simulink library "Renault_Library"  (Simulink 9.2)
settings: Solver=ode3  StartTime=0.0  StopTime=10.0  FixedStep=0.020  RelTol=1e-3  EnableMultiTasking=on
undefined symbols (not defined in the file or in its folder): ABC, AssystemProposal, b, chkval, CoeffA_C, CoeffB_C, CoeffMean_C, ConfTime_C, DiagDelay_C, DividePercent, FailureConfActive_C, FallingEdgeDuration_C, HighLevelThresholdVoltage_C, HighLevelVoltage_C, HighLevelVoltageTunable_C, InitValue_C, InputVoltRangeMax_C, InputVoltRangeMin_C, LowActiveContact_C, LowLevelThresholdVoltage_C, LowLevelVoltage_C, MaxOperatingVoltage_C, MinOperatingVoltage_C, mV2V_conversion, OUT1, OutOfRangeHigh_C, OutOfRangeLow_C, PinSupplySaturationMax, PinSupplySaturationMin, Res1Value_C, Res2Value_C, Res3Value_C, RisingEdgeDuration_C, RSA_CANSignalFailMonitoring, SampleTime_C, service14Table, service19Table, service22Table, service24Table, service28Table, service2ETable, service2FTable, service31Table, service87Table, SizeXStateDID2FTable, SizeXTable14, SizeXTable19, SizeXTable22, SizeXTable24, SizeXTable28, SizeXTable2E, SizeXTable2F, SizeXTable31, SizeXTable87, SizeYStateDID2FTable, SizeYTable14, SizeYTable19, SizeYTable22, SizeYTable24, SizeYTable28 ... (+22)
undefined symbols (not defined in the file): BatteryVoltage_ActivationThreshold_C, FrameStatus_AbsentValue_C, FrameStatus_ConfAbsentValue_C, MemThreshold_C, NAMEOFTHEMODEL_SAMPLE_TIME_CST, NbAbs1_C, NbConfAbs1_C, NbConfPres1_C, NbPres1_C, NOMDUSIGNAL_FaultConfTime_C, NOMDUSIGNAL_FaultRehabTime_C, NOMDUSIGNAL_switchvalue_C, NOMDUSIGNAL_unavailablevalue_C, PeriodFrame1_C, ResetTime_C, WakeUpTime_C
libraries: $bdroot x112, simulink x75
(part 23 of 32)

== system Renault_Library/Services ECU/Diag/RSA_DiagnosticManagement_v02.00/DIAGNOSTIC_SANCTION_TOP/Service27/SubFunction_Supported_Service27 ==
  DIAG_BUFFER_REQUEST : Inport
  BitwiseOperator1 : simulink/Logic and Bit Operations/Bitwise Operator  logicop=AND  UseBitMask=on  BitMask=bin2dec('01111111')  BitMaskRealWorld=Stored Integer
  BitwiseOperator4 : simulink/Logic and Bit Operations/Bitwise Operator  logicop=AND  UseBitMask=on  BitMask=bin2dec('10000000')  BitMaskRealWorld=Stored Integer
  Constant : Constant  OutDataTypeStr=Inherit: Inherit via back propagation  SampleTime=-1
  Constant1 : Constant  Value=2  OutDataTypeStr=Inherit: Inherit via back propagation  SampleTime=-1
  Constant10 : Constant  Value=RequestSeed5  OutDataTypeStr=Inherit: Inherit via back propagation  SampleTime=-1
  Constant{5,7,9,11,15,18,21,26,28,30} (10 alike) : Constant  Value=true  OutDataTypeStr=boolean  SampleTime=-1
  Constant12 : Constant  Value=65  OutDataTypeStr=Inherit: Inherit via back propagation  SampleTime=-1
  Constant13 : Constant  Value=4  OutDataTypeStr=Inherit: Inherit via back propagation  SampleTime=-1
  Constant14 : Constant  Value=SendKey8  OutDataTypeStr=Inherit: Inherit via back propagation  SampleTime=-1
  Constant16 : Constant  OutDataTypeStr=Inherit: Inherit via back propagation  SampleTime=-1
  Constant17 : Constant  Value=RequestSeed7  OutDataTypeStr=Inherit: Inherit via back propagation  SampleTime=-1
  Constant19 : Constant  Value=7  OutDataTypeStr=Inherit: Inherit via back propagation  SampleTime=-1
  Constant2 : Constant  Value=3  OutDataTypeStr=Inherit: Inherit via back propagation  SampleTime=-1
  Constant20 : Constant  Value=SendKey42  OutDataTypeStr=Inherit: Inherit via back propagation  SampleTime=-1
  Constant22 : Constant  Value=66  OutDataTypeStr=Inherit: Inherit via back propagation  SampleTime=-1
  Constant23 : Constant  Value=6  OutDataTypeStr=Inherit: Inherit via back propagation  SampleTime=-1
  Constant24 : Constant  Value=RequestSeed41  OutDataTypeStr=Inherit: Inherit via back propagation  SampleTime=-1
  Constant25 : Constant  Value=8  OutDataTypeStr=Inherit: Inherit via back propagation  SampleTime=-1
  Constant27 : Constant  Value=SendKey4  OutDataTypeStr=Inherit: Inherit via back propagation  SampleTime=-1
  Constant29 : Constant  Value=SendKey6  OutDataTypeStr=Inherit: Inherit via back propagation  SampleTime=-1
  Constant3 : Constant  Value=RequestSeed  OutDataTypeStr=Inherit: Inherit via back propagation  SampleTime=-1
  Constant4 : Constant  Value=5  OutDataTypeStr=Inherit: Inherit via back propagation  SampleTime=-1
  Constant6 : Constant  Value=SendKey  OutDataTypeStr=Inherit: Inherit via back propagation  SampleTime=-1
  Constant8 : Constant  Value=RequestSeed3  OutDataTypeStr=Inherit: Inherit via back propagation  SampleTime=-1
  LogicalOperator{,1..3,5..10} (10 alike) : Logic  OutDataTypeStr=boolean
  LogicalOperator4 : Logic  Operator=OR  Inputs=10  OutDataTypeStr=boolean
  RelationalOperator{,1..20} (21 alike) : RelationalOperator  Operator===  OutDataTypeStr=boolean  RndMeth=Nearest  InputSameDT=on
  Selector : Selector  InputPortWidth=4095  IndexOptions=Index vector (dialog)  Indices=[2]  OutputSizes=1
  ShiftArithmetic3 : ArithShift  BitShiftNumber=7
  SUBFUNCTION_SUPPORTED : Outport
  SUPPRESS_POS_RES : Outport  Port=2
  wires:
  DIAG_BUFFER_REQUEST/1 -> Selector/1
  Constant/1 -> RelationalOperator/2
  Constant1/1 -> RelationalOperator1/2
  Constant2/1 -> RelationalOperator2/2
  Constant4/1 -> RelationalOperator3/2
  Constant3/1 -> RelationalOperator4/1
  Constant5/1 -> RelationalOperator4/2
  RelationalOperator/1 -> LogicalOperator/2
  RelationalOperator4/1 -> LogicalOperator/1
  Constant7/1 -> RelationalOperator5/2
  Constant6/1 -> RelationalOperator5/1
  RelationalOperator1/1 -> LogicalOperator1/2
  RelationalOperator5/1 -> LogicalOperator1/1
  Constant9/1 -> RelationalOperator6/2
  Constant8/1 -> RelationalOperator6/1
  RelationalOperator2/1 -> LogicalOperator2/2
  RelationalOperator6/1 -> LogicalOperator2/1
  Constant10/1 -> RelationalOperator7/1
  Constant11/1 -> RelationalOperator7/2
  RelationalOperator7/1 -> LogicalOperator3/1
  LogicalOperator4/1 -> SUBFUNCTION_SUPPORTED/1
  RelationalOperator3/1 -> LogicalOperator3/2
  RelationalOperator16/1 -> SUPPRESS_POS_RES/1
  BitwiseOperator4/1 -> ShiftArithmetic3/1
  ShiftArithmetic3/1 -> RelationalOperator16/1
  Selector/1 -> BitwiseOperator4/1, BitwiseOperator1/1
  Constant16/1 -> RelationalOperator16/2
  BitwiseOperator1/1 -> RelationalOperator/1, RelationalOperator1/1, RelationalOperator2/1, RelationalOperator3/1, RelationalOperator12/1, RelationalOperator8/1, RelationalOperator9/1, RelationalOperator14/1, RelationalOperator15/1, RelationalOperator10/1
  Constant19/1 -> RelationalOperator12/2
  Constant17/1 -> RelationalOperator13/1
  Constant15/1 -> RelationalOperator13/2
  RelationalOperator13/1 -> LogicalOperator6/1
  RelationalOperator12/1 -> LogicalOperator6/2
  Constant12/1 -> RelationalOperator8/2
  Constant13/1 -> RelationalOperator9/2
  Constant23/1 -> RelationalOperator14/2
  Constant25/1 -> RelationalOperator15/2
  Constant24/1 -> RelationalOperator17/1
  Constant18/1 -> RelationalOperator17/2
  RelationalOperator8/1 -> LogicalOperator5/2
  RelationalOperator17/1 -> LogicalOperator5/1
  Constant21/1 -> RelationalOperator18/2
  Constant27/1 -> RelationalOperator18/1
  RelationalOperator9/1 -> LogicalOperator7/2
  RelationalOperator18/1 -> LogicalOperator7/1
  Constant26/1 -> RelationalOperator19/2
  Constant29/1 -> RelationalOperator19/1
  RelationalOperator14/1 -> LogicalOperator8/2
  RelationalOperator19/1 -> LogicalOperator8/1
  Constant14/1 -> RelationalOperator20/1
  Constant28/1 -> RelationalOperator20/2
  RelationalOperator20/1 -> LogicalOperator9/1
  RelationalOperator15/1 -> LogicalOperator9/2
  Constant22/1 -> RelationalOperator10/2
  Constant20/1 -> RelationalOperator11/1
  Constant30/1 -> RelationalOperator11/2
  RelationalOperator11/1 -> LogicalOperator10/1
  RelationalOperator10/1 -> LogicalOperator10/2
  LogicalOperator/1 -> LogicalOperator4/1
  LogicalOperator1/1 -> LogicalOperator4/2
  LogicalOperator2/1 -> LogicalOperator4/3
  LogicalOperator3/1 -> LogicalOperator4/4
  LogicalOperator6/1 -> LogicalOperator4/5
  LogicalOperator5/1 -> LogicalOperator4/6
  LogicalOperator7/1 -> LogicalOperator4/7
  LogicalOperator8/1 -> LogicalOperator4/8
  LogicalOperator9/1 -> LogicalOperator4/9
  LogicalOperator10/1 -> LogicalOperator4/10

== system Renault_Library/Services ECU/Diag/RSA_DiagnosticManagement_v02.00/DIAGNOSTIC_SANCTION_TOP/Service28/Check_Lenght_Message_Service28 ==
  REQ_DATA_NUMBER : Inport
  Constant1 : Constant  Value=3  OutDataTypeStr=Inherit: Inherit via back propagation  SampleTime=-1
  Constant8 : Constant  Value=5  OutDataTypeStr=Inherit: Inherit via back propagation  SampleTime=-1
  LogicalOperator : Logic  OutDataTypeStr=boolean
  RelationalOperator1 : RelationalOperator  OutDataTypeStr=boolean  RndMeth=Nearest  InputSameDT=on
  RelationalOperator9 : RelationalOperator  Operator=<=  OutDataTypeStr=boolean  RndMeth=Nearest  InputSameDT=on
  LENGTH_MESSAGE_CORRECT : Outport
  wires:
  REQ_DATA_NUMBER/1 -> RelationalOperator9/1, RelationalOperator1/1
  Constant8/1 -> RelationalOperator9/2
  Constant1/1 -> RelationalOperator1/2
  RelationalOperator9/1 -> LogicalOperator/1
  RelationalOperator1/1 -> LogicalOperator/2
  LogicalOperator/1 -> LENGTH_MESSAGE_CORRECT/1

== system Renault_Library/Services ECU/Diag/RSA_DiagnosticManagement_v02.00/DIAGNOSTIC_SANCTION_TOP/Service28/Parameter_Valid_Service28 ==
  DIAG_BUFFER_REQUEST : Inport
  Parameter_service28 : Stateflow chart  TreatAsAtomicUnit=on
  PARAMETER_VALID : Outport
  wires:
  DIAG_BUFFER_REQUEST/1 -> Parameter_service28/1(DIAG_BUFFER_REQUEST)
  Parameter_service28/1(PARAMETER_VALID) -> PARAMETER_VALID/1

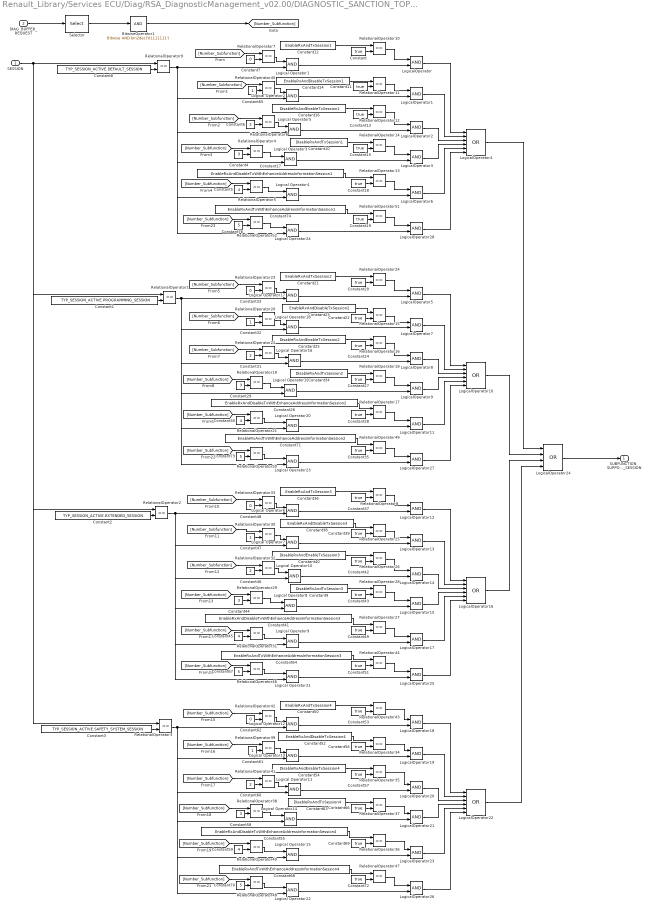
== system Renault_Library/Services ECU/Diag/RSA_DiagnosticManagement_v02.00/DIAGNOSTIC_SANCTION_TOP/Service28/SubFunction_In_Active_Session_Service28 ==
  SESSION : Inport
  DIAG_BUFFER_REQUEST : Inport  Port=2
  BitwiseOperator1 : simulink/Logic and Bit Operations/Bitwise Operator  logicop=AND  UseBitMask=on  BitMask=bin2dec('01111111')  BitMaskRealWorld=Stored Integer
  Constant{,11,13,15,18..20,22,24,27..28,35,37,39,42..43,49,51,53,56..57,66,69,72} (24 alike) : Constant  Value=true  OutDataTypeStr=boolean  SampleTime=-1
  Constant1 : Constant  Value=TYP_SESSION_ACTIVE.PROGRAMMING_SESSION  OutDataTypeStr=Inherit: Inherit via back propagation  SampleTime=-1
  Constant10 : Constant  Value=DisableRxAndTxSession1  OutDataTypeStr=Inherit: Inherit via back propagation  SampleTime=-1
  Constant12 : Constant  Value=EnableRxAndTxSession1  OutDataTypeStr=Inherit: Inherit via back propagation  SampleTime=-1
  Constant14 : Constant  Value=EnableRxAndDisableTxSession1  OutDataTypeStr=Inherit: Inherit via back propagation  SampleTime=-1
  Constant16 : Constant  Value=DisableRxAndEnableTxSession1  OutDataTypeStr=Inherit: Inherit via back propagation  SampleTime=-1
  Constant17 : Constant  Value=EnableRxAndDisableTxWithEnhanceAddressInformationSession1  OutDataTypeStr=Inherit: Inherit via back propagation  SampleTime=-1
  Constant2 : Constant  Value=TYP_SESSION_ACTIVE.EXTENDED_SESSION  OutDataTypeStr=Inherit: Inherit via back propagation  SampleTime=-1
  Constant21 : Constant  Value=EnableRxAndTxSession2  OutDataTypeStr=Inherit: Inherit via back propagation  SampleTime=-1
  Constant23 : Constant  Value=EnableRxAndDisableTxSession2  OutDataTypeStr=Inherit: Inherit via back propagation  SampleTime=-1
  Constant25 : Constant  Value=DisableRxAndEnableTxSession2  OutDataTypeStr=Inherit: Inherit via back propagation  SampleTime=-1
  Constant26 : Constant  Value=EnableRxAndDisableTxWithEnhanceAddressInformationSession2  OutDataTypeStr=Inherit: Inherit via back propagation  SampleTime=-1
  Constant{4,29,44,58} (4 alike) : Constant  Value=3  OutDataTypeStr=Inherit: Inherit via back propagation  SampleTime=-1
  Constant3 : Constant  Value=TYP_SESSION_ACTIVE.SAFETY_SYSTEM_SESSION  OutDataTypeStr=Inherit: Inherit via back propagation  SampleTime=-1
  Constant{5,30,45,59} (4 alike) : Constant  Value=4  OutDataTypeStr=Inherit: Inherit via back propagation  SampleTime=-1
  Constant{6,31,46,60} (4 alike) : Constant  Value=2  OutDataTypeStr=Inherit: Inherit via back propagation  SampleTime=-1
  Constant{32,47,61,65} (4 alike) : Constant  OutDataTypeStr=Inherit: Inherit via back propagation  SampleTime=-1
  Constant{7,33,48,62} (4 alike) : Constant  Value=0  OutDataTypeStr=Inherit: Inherit via back propagation  SampleTime=-1
  Constant34 : Constant  Value=DisableRxAndTxSession2  OutDataTypeStr=Inherit: Inherit via back propagation  SampleTime=-1
  Constant36 : Constant  Value=EnableRxAndTxSession3  OutDataTypeStr=Inherit: Inherit via back propagation  SampleTime=-1
  Constant38 : Constant  Value=EnableRxAndDisableTxSession3  OutDataTypeStr=Inherit: Inherit via back propagation  SampleTime=-1
  Constant40 : Constant  Value=DisableRxAndEnableTxSession3  OutDataTypeStr=Inherit: Inherit via back propagation  SampleTime=-1
  Constant41 : Constant  Value=EnableRxAndDisableTxWithEnhanceAddressInformationSession3  OutDataTypeStr=Inherit: Inherit via back propagation  SampleTime=-1
  Constant50 : Constant  Value=EnableRxAndTxSession4  OutDataTypeStr=Inherit: Inherit via back propagation  SampleTime=-1
  Constant52 : Constant  Value=EnableRxAndDisableTxSession4  OutDataTypeStr=Inherit: Inherit via back propagation  SampleTime=-1
  Constant54 : Constant  Value=DisableRxAndEnableTxSession4  OutDataTypeStr=Inherit: Inherit via back propagation  SampleTime=-1
  Constant55 : Constant  Value=EnableRxAndDisableTxWithEnhanceAddressInformationSession4  OutDataTypeStr=Inherit: Inherit via back propagation  SampleTime=-1
  Constant63 : Constant  Value=DisableRxAndTxSession4  OutDataTypeStr=Inherit: Inherit via back propagation  SampleTime=-1
  Constant64 : Constant  Value=EnableRxAndTxWithEnhanceAddressInformationSession3  OutDataTypeStr=Inherit: Inherit via back propagation  SampleTime=-1
  Constant{67,70,73,76} (4 alike) : Constant  Value=5  OutDataTypeStr=Inherit: Inherit via back propagation  SampleTime=-1
  Constant68 : Constant  Value=EnableRxAndTxWithEnhanceAddressInformationSession4  OutDataTypeStr=Inherit: Inherit via back propagation  SampleTime=-1
  Constant71 : Constant  Value=EnableRxAndTxWithEnhanceAddressInformationSession2  OutDataTypeStr=Inherit: Inherit via back propagation  SampleTime=-1
  Constant74 : Constant  Value=EnableRxAndTxWithEnhanceAddressInformationSession1  OutDataTypeStr=Inherit: Inherit via back propagation  SampleTime=-1
  Constant8 : Constant  Value=TYP_SESSION_ACTIVE.DEFAULT_SESSION  OutDataTypeStr=Inherit: Inherit via back propagation  SampleTime=-1
  Constant9 : Constant  Value=DisableRxAndTxSession3  OutDataTypeStr=Inherit: Inherit via back propagation  SampleTime=-1
  Logical Operator{1..24} (24 alike) : Logic  OutDataTypeStr=boolean
  LogicalOperator{,1..3,5..9,11..15,17..21,23,25..28} (24 alike) : Logic  OutDataTypeStr=boolean
  LogicalOperator{4,10,16,22} (4 alike) : Logic  Operator=OR  Inputs=6  OutDataTypeStr=boolean
  LogicalOperator24 : Logic  Operator=OR  Inputs=4  OutDataTypeStr=boolean
  RelationalOperator{1..52} (52 alike) : RelationalOperator  Operator===  OutDataTypeStr=boolean  RndMeth=Nearest  InputSameDT=on
  Selector : Selector  InputPortWidth=4095  IndexOptions=Index vector (dialog)  Indices=[2]  OutputSizes=1
  SUBFUNCTION_SUPPORTED_IN_ACTIVE_SESSION : Outport
  wires:
  Constant8/1 -> RelationalOperator9/2
  SESSION/1 -> RelationalOperator9/1, RelationalOperator1/1, RelationalOperator2/1, RelationalOperator3/1
  Constant1/1 -> RelationalOperator1/2
  Constant2/1 -> RelationalOperator2/2
  Constant3/1 -> RelationalOperator3/2
  Selector/1 -> BitwiseOperator1/1
  DIAG_BUFFER_REQUEST/1 -> Selector/1
  Constant7/1 -> RelationalOperator7/2
  Constant12/1 -> RelationalOperator10/1
  Constant/1 -> RelationalOperator10/2
  RelationalOperator10/1 -> LogicalOperator/1
  Constant11/1 -> RelationalOperator11/2
  Constant14/1 -> RelationalOperator11/1
  RelationalOperator11/1 -> LogicalOperator1/1
  Constant13/1 -> RelationalOperator12/2
  Constant16/1 -> RelationalOperator12/1
  RelationalOperator12/1 -> LogicalOperator2/1
  Constant10/1 -> RelationalOperator14/1
  Constant15/1 -> RelationalOperator14/2
  RelationalOperator14/1 -> LogicalOperator3/1
  Constant17/1 -> RelationalOperator13/1
  Constant18/1 -> RelationalOperator13/2
  RelationalOperator13/1 -> LogicalOperator6/1
  Logical Operator2/1 -> LogicalOperator1/2
  Logical Operator1/1 -> LogicalOperator/2
  RelationalOperator9/1 -> Logical Operator1/2, Logical Operator2/2, Logical Operator3/2, Logical Operator4/2, Logical Operator5/2, Logical Operator24/2
  Constant65/1 -> RelationalOperator45/2
  RelationalOperator45/1 -> Logical Operator2/1
  Constant4/1 -> RelationalOperator4/2
  Constant5/1 -> RelationalOperator5/2
  RelationalOperator5/1 -> Logical Operator4/1
  Logical Operator4/1 -> LogicalOperator6/2
  Logical Operator3/1 -> LogicalOperator3/2
  Constant6/1 -> RelationalOperator6/2
  Logical Operator5/1 -> LogicalOperator2/2
  RelationalOperator7/1 -> Logical Operator1/1
  RelationalOperator4/1 -> Logical Operator3/1
  RelationalOperator6/1 -> Logical Operator5/1
  Constant48/1 -> RelationalOperator33/2
  Constant36/1 -> RelationalOperator8/1
  Constant37/1 -> RelationalOperator8/2
  RelationalOperator8/1 -> LogicalOperator12/1
  Constant39/1 -> RelationalOperator25/2
  Constant38/1 -> RelationalOperator25/1
  RelationalOperator25/1 -> LogicalOperator13/1
  Constant42/1 -> RelationalOperator26/2
  Constant40/1 -> RelationalOperator26/1
  RelationalOperator26/1 -> LogicalOperator14/1
  Constant9/1 -> RelationalOperator28/1
  Constant43/1 -> RelationalOperator28/2
  RelationalOperator28/1 -> LogicalOperator15/1
  Constant41/1 -> RelationalOperator27/1
  Constant49/1 -> RelationalOperator27/2
  RelationalOperator27/1 -> LogicalOperator17/1
  Logical Operator7/1 -> LogicalOperator13/2
  Logical Operator6/1 -> LogicalOperator12/2
  RelationalOperator2/1 -> Logical Operator6/2, Logical Operator7/2, Logical Operator8/2, Logical Operator9/2, Logical Operator10/2, Logical Operator21/2
  Constant47/1 -> RelationalOperator30/2
  RelationalOperator30/1 -> Logical Operator7/1
  Constant44/1 -> RelationalOperator29/2
  Constant45/1 -> RelationalOperator31/2
  RelationalOperator31/1 -> Logical Operator9/1
  Logical Operator9/1 -> LogicalOperator17/2
  Logical Operator8/1 -> LogicalOperator15/2
  Constant46/1 -> RelationalOperator32/2
  Logical Operator10/1 -> LogicalOperator14/2
  RelationalOperator33/1 -> Logical Operator6/1
  RelationalOperator29/1 -> Logical Operator8/1
  RelationalOperator32/1 -> Logical Operator10/1
  Constant62/1 -> RelationalOperator42/2
  Constant50/1 -> RelationalOperator43/1
  Constant53/1 -> RelationalOperator43/2
  RelationalOperator43/1 -> LogicalOperator18/1
  Constant56/1 -> RelationalOperator34/2
  Constant52/1 -> RelationalOperator34/1
  RelationalOperator34/1 -> LogicalOperator19/1
  Constant57/1 -> RelationalOperator35/2
  Constant54/1 -> RelationalOperator35/1
  RelationalOperator35/1 -> LogicalOperator20/1
  Constant63/1 -> RelationalOperator37/1
  Constant66/1 -> RelationalOperator37/2
  RelationalOperator37/1 -> LogicalOperator21/1
  Constant55/1 -> RelationalOperator36/1
  Constant69/1 -> RelationalOperator36/2
  RelationalOperator36/1 -> LogicalOperator23/1
  Logical Operator13/1 -> LogicalOperator19/2
  Logical Operator12/1 -> LogicalOperator18/2
  RelationalOperator3/1 -> Logical Operator13/2, Logical Operator14/2, Logical Operator15/2, Logical Operator11/2, Logical Operator12/2, Logical Operator22/2
  Constant61/1 -> RelationalOperator39/2
  RelationalOperator39/1 -> Logical Operator13/1
  Constant58/1 -> RelationalOperator38/2
  Constant59/1 -> RelationalOperator40/2
  RelationalOperator40/1 -> Logical Operator15/1
  Logical Operator15/1 -> LogicalOperator23/2
  Logical Operator14/1 -> LogicalOperator21/2
  Constant60/1 -> RelationalOperator41/2
  Logical Operator11/1 -> LogicalOperator20/2
  RelationalOperator42/1 -> Logical Operator12/1
  RelationalOperator38/1 -> Logical Operator14/1
  RelationalOperator41/1 -> Logical Operator11/1
  Constant33/1 -> RelationalOperator23/2
  Constant21/1 -> RelationalOperator24/1
  Constant20/1 -> RelationalOperator24/2
  RelationalOperator24/1 -> LogicalOperator5/1
  Constant22/1 -> RelationalOperator15/2
  Constant23/1 -> RelationalOperator15/1
  RelationalOperator15/1 -> LogicalOperator7/1
  Constant24/1 -> RelationalOperator16/2
  Constant25/1 -> RelationalOperator16/1
  RelationalOperator16/1 -> LogicalOperator8/1
  Constant34/1 -> RelationalOperator18/1
  Constant27/1 -> RelationalOperator18/2
  RelationalOperator18/1 -> LogicalOperator9/1
  Constant26/1 -> RelationalOperator17/1
  Constant28/1 -> RelationalOperator17/2
  RelationalOperator17/1 -> LogicalOperator11/1
  Logical Operator18/1 -> LogicalOperator7/2
  Logical Operator17/1 -> LogicalOperator5/2
  RelationalOperator1/1 -> Logical Operator17/2, Logical Operator18/2, Logical Operator19/2, Logical Operator20/2, Logical Operator16/2, Logical Operator23/2
  Constant32/1 -> RelationalOperator20/2
  RelationalOperator20/1 -> Logical Operator18/1
  Constant29/1 -> RelationalOperator19/2
  Constant30/1 -> RelationalOperator21/2
  RelationalOperator21/1 -> Logical Operator20/1
  Logical Operator20/1 -> LogicalOperator11/2
  Logical Operator19/1 -> LogicalOperator9/2
  Constant31/1 -> RelationalOperator22/2
  Logical Operator16/1 -> LogicalOperator8/2
  RelationalOperator23/1 -> Logical Operator17/1
  RelationalOperator19/1 -> Logical Operator19/1
  RelationalOperator22/1 -> Logical Operator16/1
  LogicalOperator10/1 -> LogicalOperator24/2
  LogicalOperator16/1 -> LogicalOperator24/3
  LogicalOperator22/1 -> LogicalOperator24/4
  LogicalOperator4/1 -> LogicalOperator24/1
  LogicalOperator24/1 -> SUBFUNCTION_SUPPORTED_IN_ACTIVE_SESSION/1
  BitwiseOperator1/1 -> [Number_Subfunction]
  [Number_Subfunction] -> RelationalOperator7/1
  [Number_Subfunction] -> RelationalOperator45/1
  [Number_Subfunction] -> RelationalOperator6/1
  [Number_Subfunction] -> RelationalOperator4/1
  [Number_Subfunction] -> RelationalOperator5/1
  [Number_Subfunction] -> RelationalOperator23/1
  [Number_Subfunction] -> RelationalOperator20/1
  [Number_Subfunction] -> RelationalOperator22/1
  [Number_Subfunction] -> RelationalOperator19/1
  [Number_Subfunction] -> RelationalOperator21/1
  [Number_Subfunction] -> RelationalOperator33/1
  [Number_Subfunction] -> RelationalOperator30/1
  [Number_Subfunction] -> RelationalOperator32/1
  [Number_Subfunction] -> RelationalOperator29/1
  [Number_Subfunction] -> RelationalOperator31/1
  [Number_Subfunction] -> RelationalOperator42/1
  [Number_Subfunction] -> RelationalOperator39/1
  [Number_Subfunction] -> RelationalOperator41/1
  [Number_Subfunction] -> RelationalOperator38/1
  [Number_Subfunction] -> RelationalOperator40/1
  Constant64/1 -> RelationalOperator44/1
  Constant51/1 -> RelationalOperator44/2
  RelationalOperator44/1 -> LogicalOperator25/1
  Constant67/1 -> RelationalOperator46/2
  RelationalOperator46/1 -> Logical Operator21/1
  Logical Operator21/1 -> LogicalOperator25/2
  [Number_Subfunction] -> RelationalOperator46/1
  Constant68/1 -> RelationalOperator47/1
  Constant72/1 -> RelationalOperator47/2
  RelationalOperator47/1 -> LogicalOperator26/1
  Constant70/1 -> RelationalOperator48/2
  RelationalOperator48/1 -> Logical Operator22/1
  Logical Operator22/1 -> LogicalOperator26/2
  [Number_Subfunction] -> RelationalOperator48/1
  Constant71/1 -> RelationalOperator49/1
  Constant35/1 -> RelationalOperator49/2
  RelationalOperator49/1 -> LogicalOperator27/1
  Constant73/1 -> RelationalOperator50/2
  RelationalOperator50/1 -> Logical Operator23/1
  Logical Operator23/1 -> LogicalOperator27/2
  [Number_Subfunction] -> RelationalOperator50/1
  Constant74/1 -> RelationalOperator51/1
  Constant19/1 -> RelationalOperator51/2
  RelationalOperator51/1 -> LogicalOperator28/1
  Constant76/1 -> RelationalOperator52/2
  RelationalOperator52/1 -> Logical Operator24/1
  Logical Operator24/1 -> LogicalOperator28/2
  [Number_Subfunction] -> RelationalOperator52/1
  LogicalOperator/1 -> LogicalOperator4/1
  LogicalOperator1/1 -> LogicalOperator4/2
  LogicalOperator2/1 -> LogicalOperator4/3
  LogicalOperator3/1 -> LogicalOperator4/4
  LogicalOperator6/1 -> LogicalOperator4/5
  LogicalOperator28/1 -> LogicalOperator4/6
  LogicalOperator5/1 -> LogicalOperator10/1
  LogicalOperator7/1 -> LogicalOperator10/2
  LogicalOperator8/1 -> LogicalOperator10/3
  LogicalOperator9/1 -> LogicalOperator10/4
  LogicalOperator11/1 -> LogicalOperator10/5
  LogicalOperator27/1 -> LogicalOperator10/6
  LogicalOperator12/1 -> LogicalOperator16/1
  LogicalOperator13/1 -> LogicalOperator16/2
  LogicalOperator14/1 -> LogicalOperator16/3
  LogicalOperator15/1 -> LogicalOperator16/4
  LogicalOperator17/1 -> LogicalOperator16/5
  LogicalOperator25/1 -> LogicalOperator16/6
  LogicalOperator18/1 -> LogicalOperator22/1
  LogicalOperator19/1 -> LogicalOperator22/2
  LogicalOperator20/1 -> LogicalOperator22/3
  LogicalOperator21/1 -> LogicalOperator22/4
  LogicalOperator23/1 -> LogicalOperator22/5
  LogicalOperator26/1 -> LogicalOperator22/6

== system Renault_Library/Services ECU/Diag/RSA_DiagnosticManagement_v02.00/DIAGNOSTIC_SANCTION_TOP/Service28/SubFunction_Supported_Service28 ==
  DIAG_BUFFER_OUTPUT : Inport
  BitwiseOperator1 : simulink/Logic and Bit Operations/Bitwise Operator  logicop=AND  UseBitMask=on  BitMask=bin2dec('01111111')  BitMaskRealWorld=Stored Integer
  BitwiseOperator4 : simulink/Logic and Bit Operations/Bitwise Operator  logicop=AND  UseBitMask=on  BitMask=bin2dec('10000000')  BitMaskRealWorld=Stored Integer
  Constant : Constant  Value=0  OutDataTypeStr=Inherit: Inherit via back propagation  SampleTime=-1
  Constant1 : Constant  OutDataTypeStr=Inherit: Inherit via back propagation  SampleTime=-1
  Constant10 : Constant  Value=DisableRxAndTx  OutDataTypeStr=Inherit: Inherit via back propagation  SampleTime=-1
  Constant{5,7,9,11,13,15} (6 alike) : Constant  Value=true  OutDataTypeStr=boolean  SampleTime=-1
  Constant12 : Constant  Value=EnableRxAndTxWithEnhanceAddressInformation  OutDataTypeStr=Inherit: Inherit via back propagation  SampleTime=-1
  Constant14 : Constant  Value=5  OutDataTypeStr=Inherit: Inherit via back propagation  SampleTime=-1
  Constant16 : Constant  OutDataTypeStr=Inherit: Inherit via back propagation  SampleTime=-1
  Constant17 : Constant  Value=EnableRxAndDisableTxWithEnhanceAddressInformation  OutDataTypeStr=Inherit: Inherit via back propagation  SampleTime=-1
  Constant19 : Constant  Value=4  OutDataTypeStr=Inherit: Inherit via back propagation  SampleTime=-1
  Constant2 : Constant  Value=2  OutDataTypeStr=Inherit: Inherit via back propagation  SampleTime=-1
  Constant3 : Constant  Value=EnableRxAndTx  OutDataTypeStr=Inherit: Inherit via back propagation  SampleTime=-1
  Constant4 : Constant  Value=3  OutDataTypeStr=Inherit: Inherit via back propagation  SampleTime=-1
  Constant6 : Constant  Value=EnableRxAndDisableTx  OutDataTypeStr=Inherit: Inherit via back propagation  SampleTime=-1
  Constant8 : Constant  Value=DisableRxAndEnableTx  OutDataTypeStr=Inherit: Inherit via back propagation  SampleTime=-1
  LogicalOperator{,1..3,5..6} (6 alike) : Logic  OutDataTypeStr=boolean
  LogicalOperator4 : Logic  Operator=OR  Inputs=6  OutDataTypeStr=boolean
  RelationalOperator{,1..9,12..13,16} (13 alike) : RelationalOperator  Operator===  OutDataTypeStr=boolean  RndMeth=Nearest  InputSameDT=on
  Selector : Selector  InputPortWidth=4095  IndexOptions=Index vector (dialog)  Indices=[2]  OutputSizes=1
  ShiftArithmetic3 : ArithShift  BitShiftNumber=7
  SUBFUNCTION_SUPPORTED : Outport
  SUPPRESS_POS_RES : Outport  Port=2
  wires:
  DIAG_BUFFER_OUTPUT/1 -> Selector/1
  Constant/1 -> RelationalOperator/2
  Constant1/1 -> RelationalOperator1/2
  Constant2/1 -> RelationalOperator2/2
  Constant4/1 -> RelationalOperator3/2
  Constant3/1 -> RelationalOperator4/1
  Constant15/1 -> RelationalOperator4/2
  RelationalOperator/1 -> LogicalOperator/2
  RelationalOperator4/1 -> LogicalOperator/1
  Constant13/1 -> RelationalOperator5/2
  Constant6/1 -> RelationalOperator5/1
  RelationalOperator1/1 -> LogicalOperator1/2
  RelationalOperator5/1 -> LogicalOperator1/1
  Constant11/1 -> RelationalOperator6/2
  Constant8/1 -> RelationalOperator6/1
  RelationalOperator2/1 -> LogicalOperator2/2
  RelationalOperator6/1 -> LogicalOperator2/1
  Constant10/1 -> RelationalOperator7/1
  Constant9/1 -> RelationalOperator7/2
  RelationalOperator7/1 -> LogicalOperator3/1
  LogicalOperator4/1 -> SUBFUNCTION_SUPPORTED/1
  RelationalOperator3/1 -> LogicalOperator3/2
  RelationalOperator16/1 -> SUPPRESS_POS_RES/1
  BitwiseOperator4/1 -> ShiftArithmetic3/1
  ShiftArithmetic3/1 -> RelationalOperator16/1
  Selector/1 -> BitwiseOperator4/1, BitwiseOperator1/1
  Constant16/1 -> RelationalOperator16/2
  BitwiseOperator1/1 -> RelationalOperator/1, RelationalOperator1/1, RelationalOperator2/1, RelationalOperator3/1, RelationalOperator12/1, RelationalOperator8/1
  Constant19/1 -> RelationalOperator12/2
  Constant17/1 -> RelationalOperator13/1
  Constant7/1 -> RelationalOperator13/2
  RelationalOperator13/1 -> LogicalOperator6/1
  RelationalOperator12/1 -> LogicalOperator6/2
  Constant14/1 -> RelationalOperator8/2
  Constant12/1 -> RelationalOperator9/1
  Constant5/1 -> RelationalOperator9/2
  RelationalOperator9/1 -> LogicalOperator5/1
  RelationalOperator8/1 -> LogicalOperator5/2
  LogicalOperator/1 -> LogicalOperator4/1
  LogicalOperator1/1 -> LogicalOperator4/2
  LogicalOperator2/1 -> LogicalOperator4/3
  LogicalOperator3/1 -> LogicalOperator4/4
  LogicalOperator6/1 -> LogicalOperator4/5
  LogicalOperator5/1 -> LogicalOperator4/6

== system Renault_Library/Services ECU/Diag/RSA_DiagnosticManagement_v02.00/DIAGNOSTIC_SANCTION_TOP/Service2E/Check_Lenght_Message_Service2E ==
  REQ_DATA_NUMBER : Inport
  Constant1 : Constant  Value=4  OutDataTypeStr=Inherit: Inherit via back propagation  SampleTime=-1
  Constant8 : Constant  Value=4094  OutDataTypeStr=Inherit: Inherit via back propagation  SampleTime=-1
  LogicalOperator : Logic  OutDataTypeStr=boolean
  RelationalOperator1 : RelationalOperator  OutDataTypeStr=boolean  RndMeth=Nearest  InputSameDT=on
  RelationalOperator9 : RelationalOperator  Operator=<  OutDataTypeStr=boolean  RndMeth=Nearest  InputSameDT=on
  LENGTH_MESSAGE_CORRECT : Outport
  wires:
  REQ_DATA_NUMBER/1 -> RelationalOperator9/1, RelationalOperator1/1
  Constant8/1 -> RelationalOperator9/2
  Constant1/1 -> RelationalOperator1/2
  RelationalOperator9/1 -> LogicalOperator/1
  RelationalOperator1/1 -> LogicalOperator/2
  LogicalOperator/1 -> LENGTH_MESSAGE_CORRECT/1

== system Renault_Library/Services ECU/Diag/RSA_DiagnosticManagement_v02.00/DIAGNOSTIC_SANCTION_TOP/Service2E/Parameter_Valid_Service2E ==
  REQ_DATA_NUMBER : Inport
  SESSION_ACTIVE : Inport  Port=2
  DIAG_BUFFER_REQUEST : Inport  Port=3
  Parameter_service2E : Stateflow chart  TreatAsAtomicUnit=on
  SECURITY_ACCESS_ACCEPTED : Outport
  PARAMETER_VALID : Outport  Port=2
  wires:
  DIAG_BUFFER_REQUEST/1 -> Parameter_service2E/1(DIAG_BUFFER_REQUEST)
  REQ_DATA_NUMBER/1 -> Parameter_service2E/2(REQ_DATA_NUMBER)
  Parameter_service2E/1(PARAMETER_VALID) -> PARAMETER_VALID/1
  Parameter_service2E/2(SECURITY_ACCESS_ACCEPTED) -> SECURITY_ACCESS_ACCEPTED/1
  SESSION_ACTIVE/1 -> Parameter_service2E/3(SESSION_ACTIVE)

== system Renault_Library/Services ECU/Diag/RSA_DiagnosticManagement_v02.00/DIAGNOSTIC_SANCTION_TOP/Service2F/Check_Lenght_Message_Service2F ==
  REQ_DATA_NUMBER : Inport
  Constant1 : Constant  Value=3  OutDataTypeStr=Inherit: Inherit via back propagation  SampleTime=-1
  Constant8 : Constant  Value=4094  OutDataTypeStr=Inherit: Inherit via back propagation  SampleTime=-1
  LogicalOperator : Logic  OutDataTypeStr=boolean
  RelationalOperator1 : RelationalOperator  OutDataTypeStr=boolean  RndMeth=Nearest  InputSameDT=on
  RelationalOperator9 : RelationalOperator  Operator=<  OutDataTypeStr=boolean  RndMeth=Nearest  InputSameDT=on
  LENGTH_MESSAGE_CORRECT : Outport
  wires:
  REQ_DATA_NUMBER/1 -> RelationalOperator9/1, RelationalOperator1/1
  Constant8/1 -> RelationalOperator9/2
  Constant1/1 -> RelationalOperator1/2
  RelationalOperator9/1 -> LogicalOperator/1
  RelationalOperator1/1 -> LogicalOperator/2
  LogicalOperator/1 -> LENGTH_MESSAGE_CORRECT/1

== system Renault_Library/Services ECU/Diag/RSA_DiagnosticManagement_v02.00/DIAGNOSTIC_SANCTION_TOP/Service2F/Parameter_Valid_Service2F ==
  SESSION_ACTIVE : Inport
  DIAG_BUFFER_REQUEST : Inport  Port=2
  Parameter_service2F : Stateflow chart  TreatAsAtomicUnit=on
  SECURITY_ACCESS_ACCEPTED : Outport
  PARAMETER_VALID : Outport  Port=2
  wires:
  DIAG_BUFFER_REQUEST/1 -> Parameter_service2F/2(DIAG_BUFFER_REQUEST)
  Parameter_service2F/2(PARAMETER_VALID) -> PARAMETER_VALID/1
  SESSION_ACTIVE/1 -> Parameter_service2F/1(SESSION_ACTIVE)
  Parameter_service2F/1(SECURITY_ACCESS_ACCEPTED) -> SECURITY_ACCESS_ACCEPTED/1

== system Renault_Library/Services ECU/Diag/RSA_DiagnosticManagement_v02.00/DIAGNOSTIC_SANCTION_TOP/Service31/Check_Lenght_Message_Service31 ==
  REQ_DATA_NUMBER : Inport
  Constant1 : Constant  Value=4  OutDataTypeStr=Inherit: Inherit via back propagation  SampleTime=-1
  Constant8 : Constant  Value=4094  OutDataTypeStr=Inherit: Inherit via back propagation  SampleTime=-1
  LogicalOperator : Logic  OutDataTypeStr=boolean
  RelationalOperator1 : RelationalOperator  OutDataTypeStr=boolean  RndMeth=Nearest  InputSameDT=on
  RelationalOperator9 : RelationalOperator  Operator=<  OutDataTypeStr=boolean  RndMeth=Nearest  InputSameDT=on
  LENGTH_MESSAGE_CORRECT : Outport
  wires:
  REQ_DATA_NUMBER/1 -> RelationalOperator9/1, RelationalOperator1/1
  Constant8/1 -> RelationalOperator9/2
  Constant1/1 -> RelationalOperator1/2
  RelationalOperator9/1 -> LogicalOperator/1
  RelationalOperator1/1 -> LogicalOperator/2
  LogicalOperator/1 -> LENGTH_MESSAGE_CORRECT/1

== system Renault_Library/Services ECU/Diag/RSA_DiagnosticManagement_v02.00/DIAGNOSTIC_SANCTION_TOP/Service31/Parameter_Valid_Service31 ==
  DIAG_BUFFER_REQUEST : Inport
  SESSION_ACTIVE : Inport  Port=2
  Parameter_service31 : Stateflow chart  TreatAsAtomicUnit=on
  SECURITY_ACCESS_ACCEPTED : Outport
  PARAMETER_VALID : Outport  Port=2
  wires:
  DIAG_BUFFER_REQUEST/1 -> Parameter_service31/1(DIAG_BUFFER_REQUEST)
  Parameter_service31/2(PARAMETER_VALID) -> PARAMETER_VALID/1
  SESSION_ACTIVE/1 -> Parameter_service31/2(SESSION_ACTIVE)
  Parameter_service31/1(SECURITY_ACCESS_ACCEPTED) -> SECURITY_ACCESS_ACCEPTED/1

== system Renault_Library/Services ECU/Diag/RSA_DiagnosticManagement_v02.00/DIAGNOSTIC_SANCTION_TOP/Service31/SubFunction_In_Active_Session_Service31 ==
  SESSION : Inport
  DIAG_BUFFER_REQUEST : Inport  Port=2
  BitwiseOperator1 : simulink/Logic and Bit Operations/Bitwise Operator  logicop=AND  UseBitMask=on  BitMask=bin2dec('01111111')  BitMaskRealWorld=Stored Integer
  Constant1 : Constant  Value=TYP_SESSION_ACTIVE.PROGRAMMING_SESSION  OutDataTypeStr=Inherit: Inherit via back propagation  SampleTime=-1
  Constant{4..5,9..11,13,15,17..20,24} (12 alike) : Constant  Value=true  OutDataTypeStr=boolean  SampleTime=-1
  Constant12 : Constant  Value=StartRoutineSession1  OutDataTypeStr=Inherit: Inherit via back propagation  SampleTime=-1
  Constant14 : Constant  Value=StopRoutineSession1  OutDataTypeStr=Inherit: Inherit via back propagation  SampleTime=-1
  Constant16 : Constant  Value=RequestRoutineResultsSession1  OutDataTypeStr=Inherit: Inherit via back propagation  SampleTime=-1
  Constant2 : Constant  Value=TYP_SESSION_ACTIVE.EXTENDED_SESSION  OutDataTypeStr=Inherit: Inherit via back propagation  SampleTime=-1
  Constant21 : Constant  Value=StartRoutineSession2  OutDataTypeStr=Inherit: Inherit via back propagation  SampleTime=-1
  Constant23 : Constant  Value=StopRoutineSession2  OutDataTypeStr=Inherit: Inherit via back propagation  SampleTime=-1
  Constant25 : Constant  Value=RequestRoutineResultsSession2  OutDataTypeStr=Inherit: Inherit via back propagation  SampleTime=-1
  Constant3 : Constant  Value=TYP_SESSION_ACTIVE.SAFETY_SYSTEM_SESSION  OutDataTypeStr=Inherit: Inherit via back propagation  SampleTime=-1
  Constant{6,31,46,60} (4 alike) : Constant  Value=3  OutDataTypeStr=Inherit: Inherit via back propagation  SampleTime=-1
  Constant{32,47,61,65} (4 alike) : Constant  Value=2  OutDataTypeStr=Inherit: Inherit via back propagation  SampleTime=-1
  Constant{7,33,48,62} (4 alike) : Constant  OutDataTypeStr=Inherit: Inherit via back propagation  SampleTime=-1
  Constant36 : Constant  Value=StartRoutineSession3  OutDataTypeStr=Inherit: Inherit via back propagation  SampleTime=-1
  Constant38 : Constant  Value=StopRoutineSession3  OutDataTypeStr=Inherit: Inherit via back propagation  SampleTime=-1
  Constant40 : Constant  Value=RequestRoutineResultsSession3  OutDataTypeStr=Inherit: Inherit via back propagation  SampleTime=-1
  Constant50 : Constant  Value=StartRoutineSession4  OutDataTypeStr=Inherit: Inherit via back propagation  SampleTime=-1
  Constant52 : Constant  Value=StopRoutineSession4  OutDataTypeStr=Inherit: Inherit via back propagation  SampleTime=-1
  Constant54 : Constant  Value=RequestRoutineResultsSession4  OutDataTypeStr=Inherit: Inherit via back propagation  SampleTime=-1
  Constant8 : Constant  Value=TYP_SESSION_ACTIVE.DEFAULT_SESSION  OutDataTypeStr=Inherit: Inherit via back propagation  SampleTime=-1
  Logical Operator{1..2,5..7,10..13,16..18} (12 alike) : Logic  OutDataTypeStr=boolean
  LogicalOperator{,1..2,5,7..8,12..14,18..20} (12 alike) : Logic  OutDataTypeStr=boolean
  LogicalOperator{4,10,16,22} (4 alike) : Logic  Operator=OR  Inputs=3  OutDataTypeStr=boolean
  LogicalOperator24 : Logic  Operator=OR  Inputs=4  OutDataTypeStr=boolean
  RelationalOperator{1..3,6..12,15..16,20,22..26,30,32..35,39,41..43,45} (28 alike) : RelationalOperator  Operator===  OutDataTypeStr=boolean  RndMeth=Nearest  InputSameDT=on
  Selector : Selector  InputPortWidth=4095  IndexOptions=Index vector (dialog)  Indices=[2]  OutputSizes=1
  SUBFUNCTION_SUPPORTED_IN_ACTIVE_SESSION : Outport
  wires:
  Constant8/1 -> RelationalOperator9/2
  SESSION/1 -> RelationalOperator9/1, RelationalOperator1/1, RelationalOperator2/1, RelationalOperator3/1
  Constant1/1 -> RelationalOperator1/2
  Constant2/1 -> RelationalOperator2/2
  Constant3/1 -> RelationalOperator3/2
  Selector/1 -> BitwiseOperator1/1
  DIAG_BUFFER_REQUEST/1 -> Selector/1
  Constant7/1 -> RelationalOperator7/2
  Constant12/1 -> RelationalOperator10/1
  Constant24/1 -> RelationalOperator10/2
  RelationalOperator10/1 -> LogicalOperator/1
  Constant20/1 -> RelationalOperator11/2
  Constant14/1 -> RelationalOperator11/1
  RelationalOperator11/1 -> LogicalOperator1/1
  Constant19/1 -> RelationalOperator12/2
  Constant16/1 -> RelationalOperator12/1
  RelationalOperator12/1 -> LogicalOperator2/1
  LogicalOperator1/1 -> LogicalOperator4/2
  LogicalOperator2/1 -> LogicalOperator4/3
  LogicalOperator/1 -> LogicalOperator4/1
  Logical Operator2/1 -> LogicalOperator1/2
  Logical Operator1/1 -> LogicalOperator/2
  RelationalOperator9/1 -> Logical Operator1/2, Logical Operator2/2, Logical Operator5/2
  Constant65/1 -> RelationalOperator45/2
  RelationalOperator45/1 -> Logical Operator2/1
  Constant6/1 -> RelationalOperator6/2
  Logical Operator5/1 -> LogicalOperator2/2
  RelationalOperator7/1 -> Logical Operator1/1
  RelationalOperator6/1 -> Logical Operator5/1
  Constant48/1 -> RelationalOperator33/2
  Constant36/1 -> RelationalOperator8/1
  Constant4/1 -> RelationalOperator8/2
  RelationalOperator8/1 -> LogicalOperator12/1
  Constant5/1 -> RelationalOperator25/2
  Constant38/1 -> RelationalOperator25/1
  RelationalOperator25/1 -> LogicalOperator13/1
  Constant13/1 -> RelationalOperator26/2
  Constant40/1 -> RelationalOperator26/1
  RelationalOperator26/1 -> LogicalOperator14/1
  LogicalOperator13/1 -> LogicalOperator16/2
  LogicalOperator14/1 -> LogicalOperator16/3
  LogicalOperator12/1 -> LogicalOperator16/1
  Logical Operator7/1 -> LogicalOperator13/2
  Logical Operator6/1 -> LogicalOperator12/2
  RelationalOperator2/1 -> Logical Operator6/2, Logical Operator7/2, Logical Operator10/2
  Constant47/1 -> RelationalOperator30/2
  RelationalOperator30/1 -> Logical Operator7/1
  Constant46/1 -> RelationalOperator32/2
  Logical Operator10/1 -> LogicalOperator14/2
  RelationalOperator33/1 -> Logical Operator6/1
  RelationalOperator32/1 -> Logical Operator10/1
  Constant62/1 -> RelationalOperator42/2
  Constant50/1 -> RelationalOperator43/1
  Constant9/1 -> RelationalOperator43/2
  RelationalOperator43/1 -> LogicalOperator18/1
  Constant10/1 -> RelationalOperator34/2
  Constant52/1 -> RelationalOperator34/1
  RelationalOperator34/1 -> LogicalOperator19/1
  Constant11/1 -> RelationalOperator35/2
  Constant54/1 -> RelationalOperator35/1
  RelationalOperator35/1 -> LogicalOperator20/1
  LogicalOperator19/1 -> LogicalOperator22/2
  LogicalOperator20/1 -> LogicalOperator22/3
  LogicalOperator18/1 -> LogicalOperator22/1
  Logical Operator13/1 -> LogicalOperator19/2
  Logical Operator12/1 -> LogicalOperator18/2
  RelationalOperator3/1 -> Logical Operator12/2, Logical Operator13/2, Logical Operator11/2
  Constant61/1 -> RelationalOperator39/2
  RelationalOperator39/1 -> Logical Operator13/1
  Constant60/1 -> RelationalOperator41/2
  Logical Operator11/1 -> LogicalOperator20/2
  RelationalOperator42/1 -> Logical Operator12/1
  RelationalOperator41/1 -> Logical Operator11/1
  Constant33/1 -> RelationalOperator23/2
  Constant21/1 -> RelationalOperator24/1
  Constant18/1 -> RelationalOperator24/2
  RelationalOperator24/1 -> LogicalOperator5/1
  Constant17/1 -> RelationalOperator15/2
  Constant23/1 -> RelationalOperator15/1
  RelationalOperator15/1 -> LogicalOperator7/1
  Constant15/1 -> RelationalOperator16/2
  Constant25/1 -> RelationalOperator16/1
  RelationalOperator16/1 -> LogicalOperator8/1
  LogicalOperator7/1 -> LogicalOperator10/2
  LogicalOperator8/1 -> LogicalOperator10/3
  LogicalOperator5/1 -> LogicalOperator10/1
  Logical Operator18/1 -> LogicalOperator7/2
  Logical Operator17/1 -> LogicalOperator5/2
  RelationalOperator1/1 -> Logical Operator17/2, Logical Operator18/2, Logical Operator16/2
  Constant32/1 -> RelationalOperator20/2
  RelationalOperator20/1 -> Logical Operator18/1
  Constant31/1 -> RelationalOperator22/2
  Logical Operator16/1 -> LogicalOperator8/2
  RelationalOperator23/1 -> Logical Operator17/1
  RelationalOperator22/1 -> Logical Operator16/1
  LogicalOperator10/1 -> LogicalOperator24/2
  LogicalOperator16/1 -> LogicalOperator24/3
  LogicalOperator22/1 -> LogicalOperator24/4
  LogicalOperator4/1 -> LogicalOperator24/1
  LogicalOperator24/1 -> SUBFUNCTION_SUPPORTED_IN_ACTIVE_SESSION/1
  BitwiseOperator1/1 -> [Number_Subfunction]
  [Number_Subfunction] -> RelationalOperator7/1
  [Number_Subfunction] -> RelationalOperator45/1
  [Number_Subfunction] -> RelationalOperator6/1
  [Number_Subfunction] -> RelationalOperator23/1
  [Number_Subfunction] -> RelationalOperator20/1
  [Number_Subfunction] -> RelationalOperator22/1
  [Number_Subfunction] -> RelationalOperator33/1
  [Number_Subfunction] -> RelationalOperator30/1
  [Number_Subfunction] -> RelationalOperator32/1
  [Number_Subfunction] -> RelationalOperator42/1
  [Number_Subfunction] -> RelationalOperator39/1
  [Number_Subfunction] -> RelationalOperator41/1

== system Renault_Library/Services ECU/Diag/RSA_DiagnosticManagement_v02.00/DIAGNOSTIC_SANCTION_TOP/Service31/SubFunction_Supported_Service31 ==
  DIAG_BUFFER_REQUEST : Inport
  BitwiseOperator1 : simulink/Logic and Bit Operations/Bitwise Operator  logicop=AND  UseBitMask=on  BitMask=bin2dec('01111111')  BitMaskRealWorld=Stored Integer
  BitwiseOperator4 : simulink/Logic and Bit Operations/Bitwise Operator  logicop=AND  UseBitMask=on  BitMask=bin2dec('10000000')  BitMaskRealWorld=Stored Integer
  Constant1 : Constant  OutDataTypeStr=Inherit: Inherit via back propagation  SampleTime=-1
  Constant10 : Constant  Value=RequestRoutineResults  OutDataTypeStr=Inherit: Inherit via back propagation  SampleTime=-1
  Constant16 : Constant  OutDataTypeStr=Inherit: Inherit via back propagation  SampleTime=-1
  Constant2 : Constant  Value=2  OutDataTypeStr=Inherit: Inherit via back propagation  SampleTime=-1
  Constant3 : Constant  Value=true  OutDataTypeStr=boolean  SampleTime=-1
  Constant4 : Constant  Value=3  OutDataTypeStr=Inherit: Inherit via back propagation  SampleTime=-1
  Constant5 : Constant  Value=true  OutDataTypeStr=boolean  SampleTime=-1
  Constant6 : Constant  Value=StartRoutine  OutDataTypeStr=Inherit: Inherit via back propagation  SampleTime=-1
  Constant7 : Constant  Value=true  OutDataTypeStr=boolean  SampleTime=-1
  Constant8 : Constant  Value=StopRoutine  OutDataTypeStr=Inherit: Inherit via back propagation  SampleTime=-1
  LogicalOperator1 : Logic  OutDataTypeStr=boolean
  LogicalOperator2 : Logic  OutDataTypeStr=boolean
  LogicalOperator3 : Logic  OutDataTypeStr=boolean
  LogicalOperator4 : Logic  Operator=OR  Inputs=3  OutDataTypeStr=boolean
  RelationalOperator{1..3,5..7,16} (7 alike) : RelationalOperator  Operator===  OutDataTypeStr=boolean  RndMeth=Nearest  InputSameDT=on
  Selector : Selector  InputPortWidth=4095  IndexOptions=Index vector (dialog)  Indices=[2]  OutputSizes=1
  ShiftArithmetic3 : ArithShift  BitShiftNumber=7
  SUBFUNCTION_SUPPORTED : Outport
  SUPPRESS_POS_RES : Outport  Port=2
  wires:
  DIAG_BUFFER_REQUEST/1 -> Selector/1
  Constant1/1 -> RelationalOperator1/2
  Constant2/1 -> RelationalOperator2/2
  Constant4/1 -> RelationalOperator3/2
  Constant7/1 -> RelationalOperator5/2
  Constant6/1 -> RelationalOperator5/1
  RelationalOperator1/1 -> LogicalOperator1/2
  RelationalOperator5/1 -> LogicalOperator1/1
  Constant5/1 -> RelationalOperator6/2
  Constant8/1 -> RelationalOperator6/1
  RelationalOperator2/1 -> LogicalOperator2/2
  RelationalOperator6/1 -> LogicalOperator2/1
  Constant10/1 -> RelationalOperator7/1
  Constant3/1 -> RelationalOperator7/2
  RelationalOperator7/1 -> LogicalOperator3/1
  LogicalOperator4/1 -> SUBFUNCTION_SUPPORTED/1
  RelationalOperator3/1 -> LogicalOperator3/2
  RelationalOperator16/1 -> SUPPRESS_POS_RES/1
  BitwiseOperator4/1 -> ShiftArithmetic3/1
  ShiftArithmetic3/1 -> RelationalOperator16/1
  Selector/1 -> BitwiseOperator4/1, BitwiseOperator1/1
  Constant16/1 -> RelationalOperator16/2
  BitwiseOperator1/1 -> RelationalOperator1/1, RelationalOperator2/1, RelationalOperator3/1
  LogicalOperator1/1 -> LogicalOperator4/1
  LogicalOperator2/1 -> LogicalOperator4/2
  LogicalOperator3/1 -> LogicalOperator4/3

== system Renault_Library/Services ECU/Diag/RSA_DiagnosticManagement_v02.00/DIAGNOSTIC_SANCTION_TOP/Service3D/Check_Lenght_Message_Service3D ==
  REQ_DATA_NUMBER : Inport
  Constant1 : Constant  Value=5  OutDataTypeStr=Inherit: Inherit via back propagation  SampleTime=-1
  Constant8 : Constant  Value=4094  OutDataTypeStr=Inherit: Inherit via back propagation  SampleTime=-1
  LogicalOperator : Logic  OutDataTypeStr=boolean
  RelationalOperator1 : RelationalOperator  OutDataTypeStr=boolean  RndMeth=Nearest  InputSameDT=on
  RelationalOperator9 : RelationalOperator  Operator=<  OutDataTypeStr=boolean  RndMeth=Nearest  InputSameDT=on
  LENGTH_MESSAGE_CORRECT : Outport
  wires:
  LogicalOperator/1 -> LENGTH_MESSAGE_CORRECT/1
  RelationalOperator1/1 -> LogicalOperator/2
  RelationalOperator9/1 -> LogicalOperator/1
  Constant1/1 -> RelationalOperator1/2
  Constant8/1 -> RelationalOperator9/2
  REQ_DATA_NUMBER/1 -> RelationalOperator1/1, RelationalOperator9/1

== system Renault_Library/Services ECU/Diag/RSA_DiagnosticManagement_v02.00/DIAGNOSTIC_SANCTION_TOP/Service3D/Parameter_Valid_Service3D ==
  REQ_DATA_NUMBER : Inport
  SESSION_ACTIVE : Inport  Port=2
  DIAG_BUFFER_REQUEST : Inport  Port=3
  Parameter_service3D : Stateflow chart  TreatAsAtomicUnit=on
  SECURITY_ACCESS_ACCEPTED : Outport
  PARAMETER_VALID : Outport  Port=2
  wires:
  SESSION_ACTIVE/1 -> Parameter_service3D/3(SESSION_ACTIVE)
  Parameter_service3D/2(SECURITY_ACCESS_ACCEPTED) -> SECURITY_ACCESS_ACCEPTED/1
  Parameter_service3D/1(PARAMETER_VALID) -> PARAMETER_VALID/1
  REQ_DATA_NUMBER/1 -> Parameter_service3D/2(REQ_DATA_NUMBER)
  DIAG_BUFFER_REQUEST/1 -> Parameter_service3D/1(DIAG_BUFFER_REQUEST)

== system Renault_Library/Services ECU/Diag/RSA_DiagnosticManagement_v02.00/DIAGNOSTIC_SANCTION_TOP/Service3E/Check_Lenght_Message_Service3E ==
  REQ_DATA_NUMBER : Inport
  Constant1 : Constant  Value=2  OutDataTypeStr=Inherit: Inherit via back propagation  SampleTime=-1
  RelationalOperator1 : RelationalOperator  Operator===  OutDataTypeStr=boolean  RndMeth=Nearest  InputSameDT=on
  LENGTH_MESSAGE_CORRECT : Outport
  wires:
  REQ_DATA_NUMBER/1 -> RelationalOperator1/1
  Constant1/1 -> RelationalOperator1/2
  RelationalOperator1/1 -> LENGTH_MESSAGE_CORRECT/1
